simulink model "v13_aa_rii_16r128dr_ver104"  (Simulink 7.9)
settings: Solver=FixedStepDiscrete  SolverMode=SingleTasking  StartTime=0.0  StopTime=32768  RelTol=1e-3
undefined symbols (not defined in the file or in its folder): sfp
libraries: xbsIndex_r4 x19795, xps_library x54, casper_library_misc x36, dspsigops x32
(part 4 of 212)

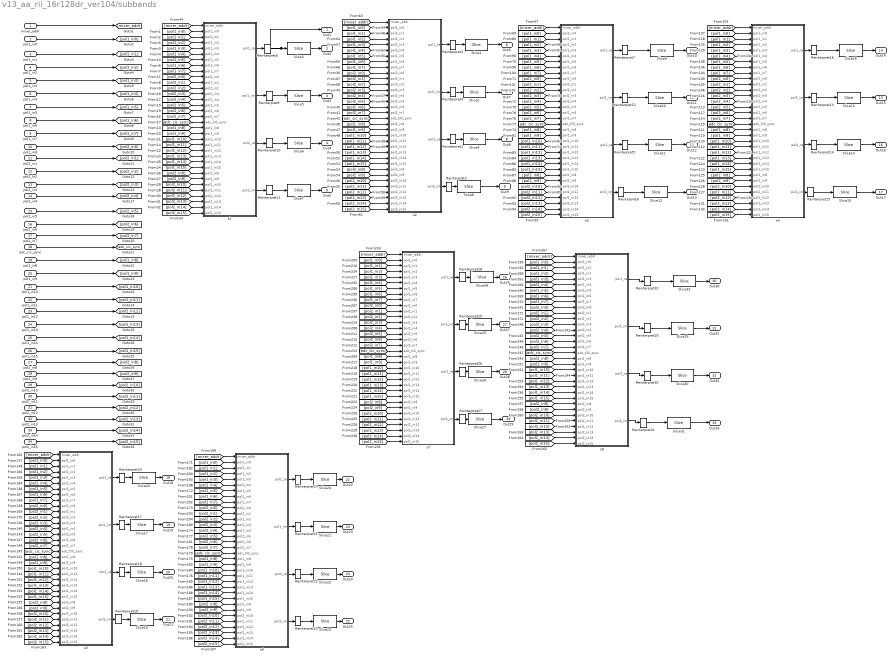
== system v13_aa_rii_16r128dr_ver104/subbands ==
  mixer_addr : Inport
  pol1_in0 : Inport  Port=2
  pol1_in1 : Inport  Port=3
  pol1_in2 : Inport  Port=4
  pol1_in3 : Inport  Port=5
  pol1_in4 : Inport  Port=6
  pol1_in5 : Inport  Port=7
  pol1_in6 : Inport  Port=8
  pol1_in7 : Inport  Port=9
  pol2_in0 : Inport  Port=10
  pol2_in1 : Inport  Port=11
  pol2_In2 : Inport  Port=12
  pol2_in3 : Inport  Port=13
  pol2_in4 : Inport  Port=14
  pol2_in5 : Inport  Port=15
  pol2_in6 : Inport  Port=16
  pol2_in7 : Inport  Port=17
  adc_cic_sync : Inport  Port=18
  pol1_in8 : Inport  Port=19
  pol1_in9 : Inport  Port=20
  pol1_in10 : Inport  Port=21
  pol1_in11 : Inport  Port=22
  pol1_in12 : Inport  Port=23
  pol1_in13 : Inport  Port=24
  pol1_in14 : Inport  Port=25
  pol1_in15 : Inport  Port=26
  pol2_in8 : Inport  Port=27
  pol2_in9 : Inport  Port=28
  pol2_in10 : Inport  Port=29
  pol2_in11 : Inport  Port=30
  pol2_in12 : Inport  Port=31
  pol2_in13 : Inport  Port=32
  pol2_in14 : Inport  Port=33
  pol2_in15 : Inport  Port=34
  Reinterpret{1..32} (32 alike) : xbsIndex_r4/Reinterpret  force_arith_type=on  arith_type=Unsigned  force_bin_pt=on  bin_pt=0  block_type=reinterpret
  Slice{1..32} (32 alike) : xbsIndex_r4/Slice  nbits=8  boolean_output=off  mode=Upper Bit Location + Width  bit1=0  base1=MSB of Input  bit0=0  base0=LSB of Input  dbl_ovrd=off  block_type=slice
  s1 : Subsystem  mask{mult_latency=5, add_latency=2, bram_length=10}
      mask prompts: mult_latency "Multiplier Latency"; add_latency "Adder Latency"; bram_length "BRAM Length(2^n)"
  s2 : Subsystem  mask{mult_latency=5, add_latency=2, bram_length=10}
      mask prompts: mult_latency "Multiplier Latency"; add_latency "Adder Latency"; bram_length "BRAM Length(2^n)"
  s3 : Subsystem  mask{mult_latency=5, add_latency=2, bram_length=10}
      mask prompts: mult_latency "Multiplier Latency"; add_latency "Adder Latency"; bram_length "BRAM Length(2^n)"
  s4 : Subsystem  mask{mult_latency=5, add_latency=2, bram_length=10}
      mask prompts: mult_latency "Multiplier Latency"; add_latency "Adder Latency"; bram_length "BRAM Length(2^n)"
  s5 : Subsystem  mask{mult_latency=5, add_latency=2, bram_length=10}
      mask prompts: mult_latency "Multiplier Latency"; add_latency "Adder Latency"; bram_length "BRAM Length(2^n)"
  s6 : Subsystem  mask{mult_latency=5, add_latency=2, bram_length=10}
      mask prompts: mult_latency "Multiplier Latency"; add_latency "Adder Latency"; bram_length "BRAM Length(2^n)"
  s7 : Subsystem  mask{mult_latency=5, add_latency=2, bram_length=10}
      mask prompts: mult_latency "Multiplier Latency"; add_latency "Adder Latency"; bram_length "BRAM Length(2^n)"
  s8 : Subsystem  mask{mult_latency=5, add_latency=2, bram_length=10}
      mask prompts: mult_latency "Multiplier Latency"; add_latency "Adder Latency"; bram_length "BRAM Length(2^n)"
  Out1 : Outport
  Out2 : Outport  Port=2
  Out3 : Outport  Port=3
  Out4 : Outport  Port=4
  Out5 : Outport  Port=5
  Out6 : Outport  Port=6
  Out7 : Outport  Port=7
  Out8 : Outport  Port=8
  Out9 : Outport  Port=9
  Out10 : Outport  Port=10
  Out11 : Outport  Port=11
  Out12 : Outport  Port=12
  Out13 : Outport  Port=13
  Out14 : Outport  Port=14
  Out15 : Outport  Port=15
  Out16 : Outport  Port=16
  Out17 : Outport  Port=17
  Out18 : Outport  Port=18
  Out19 : Outport  Port=19
  Out20 : Outport  Port=20
  Out21 : Outport  Port=21
  Out22 : Outport  Port=22
  Out23 : Outport  Port=23
  Out24 : Outport  Port=24
  Out25 : Outport  Port=25
  Out26 : Outport  Port=26
  Out27 : Outport  Port=27
  Out28 : Outport  Port=28
  Out29 : Outport  Port=29
  Out30 : Outport  Port=30
  Out31 : Outport  Port=31
  Out32 : Outport  Port=32
  Out33 : Outport  Port=33
  wires:
  Reinterpret8/1 -> Slice3/1, Out1/1
  Reinterpret9/1 -> Slice5/1
  Reinterpret10/1 -> Slice6/1
  Reinterpret11/1 -> Slice7/1
  s1/1(pol1_re) -> Reinterpret8/1
  s1/2(pol1_im) -> Reinterpret9/1
  s1/3(pol2_re) -> Reinterpret10/1
  s1/4(pol2_im) -> Reinterpret11/1
  Slice3/1 -> Out2/1
  Slice5/1 -> Out3/1
  Slice6/1 -> Out4/1
  Slice7/1 -> Out5/1
  Reinterpret3/1 -> Slice1/1
  Reinterpret4/1 -> Slice2/1
  Reinterpret1/1 -> Slice4/1
  Reinterpret2/1 -> Slice8/1
  s2/1(pol1_re) -> Reinterpret3/1
  s2/2(pol1_im) -> Reinterpret4/1
  s2/3(pol2_re) -> Reinterpret1/1
  s2/4(pol2_im) -> Reinterpret2/1
  Slice8/1 -> Out9/1
  Slice1/1 -> Out6/1
  Slice2/1 -> Out7/1
  Slice4/1 -> Out8/1
  Reinterpret7/1 -> Slice9/1
  Reinterpret12/1 -> Slice10/1
  Reinterpret5/1 -> Slice11/1
  Reinterpret6/1 -> Slice12/1
  s3/1(pol1_re) -> Reinterpret7/1
  s3/2(pol1_im) -> Reinterpret12/1
  s3/3(pol2_re) -> Reinterpret5/1
  s3/4(pol2_im) -> Reinterpret6/1
  Slice12/1 -> Out13/1
  Slice9/1 -> Out10/1
  Slice10/1 -> Out11/1
  Slice11/1 -> Out12/1
  Reinterpret16/1 -> Slice16/1
  Reinterpret13/1 -> Slice13/1
  Reinterpret14/1 -> Slice14/1
  Reinterpret15/1 -> Slice15/1
  s4/1(pol1_re) -> Reinterpret16/1
  s4/2(pol1_im) -> Reinterpret13/1
  s4/3(pol2_re) -> Reinterpret14/1
  s4/4(pol2_im) -> Reinterpret15/1
  Slice15/1 -> Out17/1
  Slice16/1 -> Out14/1
  Slice13/1 -> Out15/1
  Slice14/1 -> Out16/1
  Reinterpret20/1 -> Slice20/1
  Reinterpret17/1 -> Slice17/1
  Reinterpret18/1 -> Slice18/1
  Reinterpret19/1 -> Slice19/1
  s5/1(pol1_re) -> Reinterpret20/1
  s5/2(pol1_im) -> Reinterpret17/1
  s5/3(pol2_re) -> Reinterpret18/1
  s5/4(pol2_im) -> Reinterpret19/1
  Slice19/1 -> Out21/1
  Slice20/1 -> Out18/1
  Slice17/1 -> Out19/1
  Slice18/1 -> Out20/1
  Reinterpret24/1 -> Slice24/1
  Reinterpret21/1 -> Slice21/1
  Reinterpret22/1 -> Slice22/1
  Reinterpret23/1 -> Slice23/1
  s6/1(pol1_re) -> Reinterpret24/1
  s6/2(pol1_im) -> Reinterpret21/1
  s6/3(pol2_re) -> Reinterpret22/1
  s6/4(pol2_im) -> Reinterpret23/1
  Slice23/1 -> Out25/1
  Slice24/1 -> Out22/1
  Slice21/1 -> Out23/1
  Slice22/1 -> Out24/1
  Reinterpret28/1 -> Slice28/1
  Reinterpret25/1 -> Slice25/1
  Reinterpret26/1 -> Slice26/1
  Reinterpret27/1 -> Slice27/1
  s7/1(pol1_re) -> Reinterpret28/1
  s7/2(pol1_im) -> Reinterpret25/1
  s7/3(pol2_re) -> Reinterpret26/1
  s7/4(pol2_im) -> Reinterpret27/1
  Slice27/1 -> Out29/1
  Slice28/1 -> Out26/1
  Slice25/1 -> Out27/1
  Slice26/1 -> Out28/1
  Reinterpret32/1 -> Slice32/1
  Reinterpret29/1 -> Slice29/1
  Reinterpret30/1 -> Slice30/1
  Reinterpret31/1 -> Slice31/1
  s8/1(pol1_re) -> Reinterpret32/1
  s8/2(pol1_im) -> Reinterpret29/1
  s8/3(pol2_re) -> Reinterpret30/1
  s8/4(pol2_im) -> Reinterpret31/1
  Slice31/1 -> Out33/1
  Slice32/1 -> Out30/1
  Slice29/1 -> Out31/1
  Slice30/1 -> Out32/1
  mixer_addr/1 -> [mixer_addr]
  pol1_in0/1 -> [pol1_in0]
  pol1_in1/1 -> [pol1_in1]
  pol1_in2/1 -> [pol1_in2]
  pol1_in3/1 -> [pol1_in3]
  pol1_in4/1 -> [pol1_in4]
  pol1_in5/1 -> [pol1_in5]
  pol1_in6/1 -> [pol1_in6]
  pol1_in7/1 -> [pol1_in7]
  pol2_in0/1 -> [pol2_in0]
  pol2_in1/1 -> [pol2_in1]
  pol2_In2/1 -> [pol2_in2]
  pol2_in3/1 -> [pol2_in3]
  pol2_in4/1 -> [pol2_in4]
  pol2_in5/1 -> [pol2_in5]
  pol2_in6/1 -> [pol2_in6]
  pol2_in7/1 -> [pol2_in7]
  adc_cic_sync/1 -> [adc_cic_sync]
  pol1_in8/1 -> [pol1_in8]
  pol1_in9/1 -> [pol1_in9]
  pol1_in10/1 -> [pol1_in10]
  pol1_in11/1 -> [pol1_in11]
  pol1_in12/1 -> [pol1_in12]
  pol1_in13/1 -> [pol1_in13]
  pol1_in14/1 -> [pol1_in14]
  pol1_in15/1 -> [pol1_in15]
  pol2_in8/1 -> [pol2_in8]
  pol2_in9/1 -> [pol2_in9]
  pol2_in10/1 -> [pol2_in10]
  pol2_in11/1 -> [pol2_in11]
  pol2_in12/1 -> [pol2_in12]
  pol2_in13/1 -> [pol2_in13]
  pol2_in14/1 -> [pol2_in14]
  pol2_in15/1 -> [pol2_in15]
  [mixer_addr] -> s1/1(mixer_addr)
  [pol1_in0] -> s1/2(pol1_in0)
  [pol1_in1] -> s1/3(pol1_in1)
  [pol1_in2] -> s1/4(pol1_in2)
  [pol1_in3] -> s1/5(pol1_in3)
  [pol1_in4] -> s1/6(pol1_in4)
  [pol1_in5] -> s1/7(pol1_in5)
  [pol1_in6] -> s1/8(pol1_in6)
  [pol1_in7] -> s1/9(pol1_in7)
  [pol2_in0] -> s1/10(pol2_in0)
  [pol2_in1] -> s1/11(pol2_in1)
  [pol2_in2] -> s1/12(pol2_in2)
  [pol2_in3] -> s1/13(pol2_in3)
  [pol2_in4] -> s1/14(pol2_in4)
  [pol2_in5] -> s1/15(pol2_in5)
  [pol2_in6] -> s1/16(pol2_in6)
  [pol2_in7] -> s1/17(pol2_in7)
  [adc_cic_sync] -> s1/18(adc_CIC_sync)
  [pol1_in8] -> s1/19(pol1_in8)
  [pol1_in9] -> s1/20(pol1_in9)
  [pol1_in10] -> s1/21(pol1_in10)
  [pol1_in11] -> s1/22(pol1_in11)
  [pol1_in12] -> s1/23(pol1_in12)
  [pol1_in13] -> s1/24(pol1_in13)
  [pol1_in14] -> s1/25(pol1_in14)
  [pol1_in15] -> s1/26(pol1_in15)
  [pol2_in8] -> s1/27(pol2_in8)
  [pol2_in9] -> s1/28(pol2_in9)
  [pol2_in10] -> s1/29(pol2_in10)
  [pol2_in11] -> s1/30(pol2_in11)
  [pol2_in12] -> s1/31(pol2_in12)
  [pol2_in13] -> s1/32(pol2_in13)
  [pol2_in14] -> s1/33(pol2_in14)
  [pol2_in15] -> s1/34(pol2_in15)
  [mixer_addr] -> s2/1(mixer_addr)
  [pol1_in0] -> s2/2(pol1_in0)
  [pol1_in1] -> s2/3(pol1_in1)
  [pol1_in2] -> s2/4(pol1_in2)
  [pol1_in3] -> s2/5(pol1_in3)
  [pol1_in4] -> s2/6(pol1_in4)
  [pol1_in5] -> s2/7(pol1_in5)
  [pol1_in6] -> s2/8(pol1_in6)
  [pol1_in7] -> s2/9(pol1_in7)
  [pol2_in0] -> s2/10(pol2_in0)
  [pol2_in1] -> s2/11(pol2_in1)
  [pol2_in2] -> s2/12(pol2_in2)
  [pol2_in3] -> s2/13(pol2_in3)
  [pol2_in4] -> s2/14(pol2_in4)
  [pol2_in5] -> s2/15(pol2_in5)
  [pol2_in6] -> s2/16(pol2_in6)
  [pol2_in7] -> s2/17(pol2_in7)
  [adc_cic_sync] -> s2/18(adc_CIC_sync)
  [pol1_in8] -> s2/19(pol1_in8)
  [pol1_in9] -> s2/20(pol1_in9)
  [pol1_in10] -> s2/21(pol1_in10)
  [pol1_in11] -> s2/22(pol1_in11)
  [pol1_in12] -> s2/23(pol1_in12)
  [pol1_in13] -> s2/24(pol1_in13)
  [pol1_in14] -> s2/25(pol1_in14)
  [pol1_in15] -> s2/26(pol1_in15)
  [pol2_in8] -> s2/27(pol2_in8)
  [pol2_in9] -> s2/28(pol2_in9)
  [pol2_in10] -> s2/29(pol2_in10)
  [pol2_in11] -> s2/30(pol2_in11)
  [pol2_in12] -> s2/31(pol2_in12)
  [pol2_in13] -> s2/32(pol2_in13)
  [pol2_in14] -> s2/33(pol2_in14)
  [pol2_in15] -> s2/34(pol2_in15)
  [mixer_addr] -> s3/1(mixer_addr)
  [pol1_in0] -> s3/2(pol1_in0)
  [pol1_in1] -> s3/3(pol1_in1)
  [pol1_in2] -> s3/4(pol1_in2)
  [pol1_in3] -> s3/5(pol1_in3)
  [pol1_in4] -> s3/6(pol1_in4)
  [pol1_in5] -> s3/7(pol1_in5)
  [pol1_in6] -> s3/8(pol1_in6)
  [pol1_in7] -> s3/9(pol1_in7)
  [pol2_in0] -> s3/10(pol2_in0)
  [pol2_in1] -> s3/11(pol2_in1)
  [pol2_in2] -> s3/12(pol2_in2)
  [pol2_in3] -> s3/13(pol2_in3)
  [pol2_in4] -> s3/14(pol2_in4)
  [pol2_in5] -> s3/15(pol2_in5)
  [pol2_in6] -> s3/16(pol2_in6)
  [pol2_in7] -> s3/17(pol2_in7)
  [adc_cic_sync] -> s3/18(adc_CIC_sync)
  [pol1_in8] -> s3/19(pol1_in8)
  [pol1_in9] -> s3/20(pol1_in9)
  [pol1_in10] -> s3/21(pol1_in10)
  [pol1_in11] -> s3/22(pol1_in11)
  [pol1_in12] -> s3/23(pol1_in12)
  [pol1_in13] -> s3/24(pol1_in13)
  [pol1_in14] -> s3/25(pol1_in14)
  [pol1_in15] -> s3/26(pol1_in15)
  [pol2_in8] -> s3/27(pol2_in8)
  [pol2_in9] -> s3/28(pol2_in9)
  [pol2_in10] -> s3/29(pol2_in10)
  [pol2_in11] -> s3/30(pol2_in11)
  [pol2_in12] -> s3/31(pol2_in12)
  [pol2_in13] -> s3/32(pol2_in13)
  [pol2_in14] -> s3/33(pol2_in14)
  [pol2_in15] -> s3/34(pol2_in15)
  [mixer_addr] -> s4/1(mixer_addr)
  [pol1_in0] -> s4/2(pol1_in0)
  [pol1_in1] -> s4/3(pol1_in1)
  [pol1_in2] -> s4/4(pol1_in2)
  [pol1_in3] -> s4/5(pol1_in3)
  [pol1_in4] -> s4/6(pol1_in4)
  [pol1_in5] -> s4/7(pol1_in5)
  [pol1_in6] -> s4/8(pol1_in6)
  [pol1_in7] -> s4/9(pol1_in7)
  [pol2_in0] -> s4/10(pol2_in0)
  [pol2_in1] -> s4/11(pol2_in1)
  [pol2_in2] -> s4/12(pol2_in2)
  [pol2_in3] -> s4/13(pol2_in3)
  [pol2_in4] -> s4/14(pol2_in4)
  [pol2_in5] -> s4/15(pol2_in5)
  [pol2_in6] -> s4/16(pol2_in6)
  [pol2_in7] -> s4/17(pol2_in7)
  [adc_cic_sync] -> s4/18(adc_CIC_sync)
  [pol1_in8] -> s4/19(pol1_in8)
  [pol1_in9] -> s4/20(pol1_in9)
  [pol1_in10] -> s4/21(pol1_in10)
  [pol1_in11] -> s4/22(pol1_in11)
  [pol1_in12] -> s4/23(pol1_in12)
  [pol1_in13] -> s4/24(pol1_in13)
  [pol1_in14] -> s4/25(pol1_in14)
  [pol1_in15] -> s4/26(pol1_in15)
  [pol2_in8] -> s4/27(pol2_in8)
  [pol2_in9] -> s4/28(pol2_in9)
  [pol2_in10] -> s4/29(pol2_in10)
  [pol2_in11] -> s4/30(pol2_in11)
  [pol2_in12] -> s4/31(pol2_in12)
  [pol2_in13] -> s4/32(pol2_in13)
  [pol2_in14] -> s4/33(pol2_in14)
  [pol2_in15] -> s4/34(pol2_in15)
  [mixer_addr] -> s5/1(mixer_addr)
  [pol1_in0] -> s5/2(pol1_in0)
  [pol1_in1] -> s5/3(pol1_in1)
  [pol1_in2] -> s5/4(pol1_in2)
  [pol1_in3] -> s5/5(pol1_in3)
  [pol1_in4] -> s5/6(pol1_in4)
  [pol1_in5] -> s5/7(pol1_in5)
  [pol1_in6] -> s5/8(pol1_in6)
  [pol1_in7] -> s5/9(pol1_in7)
  [pol2_in0] -> s5/10(pol2_in0)
  [pol2_in1] -> s5/11(pol2_in1)
  [pol2_in2] -> s5/12(pol2_in2)
  [pol2_in3] -> s5/13(pol2_in3)
  [pol2_in4] -> s5/14(pol2_in4)
  [pol2_in5] -> s5/15(pol2_in5)
  [pol2_in6] -> s5/16(pol2_in6)
  [pol2_in7] -> s5/17(pol2_in7)
  [adc_cic_sync] -> s5/18(adc_CIC_sync)
  [pol1_in8] -> s5/19(pol1_in8)
  [pol1_in9] -> s5/20(pol1_in9)
  [pol1_in10] -> s5/21(pol1_in10)
  [pol1_in11] -> s5/22(pol1_in11)
  [pol1_in12] -> s5/23(pol1_in12)
  [pol1_in13] -> s5/24(pol1_in13)
  [pol1_in14] -> s5/25(pol1_in14)
  [pol1_in15] -> s5/26(pol1_in15)
  [pol2_in8] -> s5/27(pol2_in8)
  [pol2_in9] -> s5/28(pol2_in9)
  [pol2_in10] -> s5/29(pol2_in10)
  [pol2_in11] -> s5/30(pol2_in11)
  [pol2_in12] -> s5/31(pol2_in12)
  [pol2_in13] -> s5/32(pol2_in13)
  [pol2_in14] -> s5/33(pol2_in14)
  [pol2_in15] -> s5/34(pol2_in15)
  [mixer_addr] -> s8/1(mixer_addr)
  [mixer_addr] -> s7/1(mixer_addr)
  [mixer_addr] -> s6/1(mixer_addr)
  [pol1_in0] -> s6/2(pol1_in0)
  [pol1_in1] -> s6/3(pol1_in1)
  [pol1_in2] -> s6/4(pol1_in2)
  [pol1_in3] -> s6/5(pol1_in3)
  [pol1_in4] -> s6/6(pol1_in4)
  [pol1_in5] -> s6/7(pol1_in5)
  [pol1_in6] -> s6/8(pol1_in6)
  [pol1_in7] -> s6/9(pol1_in7)
  [pol2_in0] -> s6/10(pol2_in0)
  [pol2_in1] -> s6/11(pol2_in1)
  [pol2_in2] -> s6/12(pol2_in2)
  [pol2_in3] -> s6/13(pol2_in3)
  [pol2_in4] -> s6/14(pol2_in4)
  [pol2_in5] -> s6/15(pol2_in5)
  [pol2_in6] -> s6/16(pol2_in6)
  [pol2_in7] -> s6/17(pol2_in7)
  [adc_cic_sync] -> s6/18(adc_CIC_sync)
  [pol1_in8] -> s6/19(pol1_in8)
  [pol1_in9] -> s6/20(pol1_in9)
  [pol1_in10] -> s6/21(pol1_in10)
  [pol1_in11] -> s6/22(pol1_in11)
  [pol1_in12] -> s6/23(pol1_in12)
  [pol1_in13] -> s6/24(pol1_in13)
  [pol1_in14] -> s6/25(pol1_in14)
  [pol1_in15] -> s6/26(pol1_in15)
  [pol2_in8] -> s6/27(pol2_in8)
  [pol2_in9] -> s6/28(pol2_in9)
  [pol2_in10] -> s6/29(pol2_in10)
  [pol2_in11] -> s6/30(pol2_in11)
  [pol2_in12] -> s6/31(pol2_in12)
  [pol2_in13] -> s6/32(pol2_in13)
  [pol2_in14] -> s6/33(pol2_in14)
  [pol2_in15] -> s6/34(pol2_in15)
  [pol1_in0] -> s7/2(pol1_in0)
  [pol1_in1] -> s7/3(pol1_in1)
  [pol1_in2] -> s7/4(pol1_in2)
  [pol1_in3] -> s7/5(pol1_in3)
  [pol1_in4] -> s7/6(pol1_in4)
  [pol1_in5] -> s7/7(pol1_in5)
  [pol1_in6] -> s7/8(pol1_in6)
  [pol1_in7] -> s7/9(pol1_in7)
  [pol2_in0] -> s7/10(pol2_in0)
  [pol2_in1] -> s7/11(pol2_in1)
  [pol2_in2] -> s7/12(pol2_in2)
  [pol2_in3] -> s7/13(pol2_in3)
  [pol2_in4] -> s7/14(pol2_in4)
  [pol2_in5] -> s7/15(pol2_in5)
  [pol2_in6] -> s7/16(pol2_in6)
  [pol2_in7] -> s7/17(pol2_in7)
  [adc_cic_sync] -> s7/18(adc_CIC_sync)
  [pol1_in8] -> s7/19(pol1_in8)
  [pol1_in9] -> s7/20(pol1_in9)
  [pol1_in10] -> s7/21(pol1_in10)
  [pol1_in11] -> s7/22(pol1_in11)
  [pol1_in12] -> s7/23(pol1_in12)
  [pol1_in13] -> s7/24(pol1_in13)
  [pol1_in14] -> s7/25(pol1_in14)
  [pol1_in15] -> s7/26(pol1_in15)
  [pol2_in8] -> s7/27(pol2_in8)
  [pol2_in9] -> s7/28(pol2_in9)
  [pol2_in10] -> s7/29(pol2_in10)
  [pol2_in11] -> s7/30(pol2_in11)
  [pol2_in12] -> s7/31(pol2_in12)
  [pol2_in13] -> s7/32(pol2_in13)
  [pol2_in14] -> s7/33(pol2_in14)
  [pol2_in15] -> s7/34(pol2_in15)
  [pol1_in0] -> s8/2(pol1_in0)
  [pol1_in1] -> s8/3(pol1_in1)
  [pol1_in2] -> s8/4(pol1_in2)
  [pol1_in3] -> s8/5(pol1_in3)
  [pol1_in4] -> s8/6(pol1_in4)
  [pol1_in5] -> s8/7(pol1_in5)
  [pol1_in6] -> s8/8(pol1_in6)
  [pol1_in7] -> s8/9(pol1_in7)
  [pol2_in0] -> s8/10(pol2_in0)
  [pol2_in1] -> s8/11(pol2_in1)
  [pol2_in2] -> s8/12(pol2_in2)
  [pol2_in3] -> s8/13(pol2_in3)
  [pol2_in4] -> s8/14(pol2_in4)
  [pol2_in5] -> s8/15(pol2_in5)
  [pol2_in6] -> s8/16(pol2_in6)
  [pol2_in7] -> s8/17(pol2_in7)
  [adc_cic_sync] -> s8/18(adc_CIC_sync)
  [pol1_in8] -> s8/19(pol1_in8)
  [pol1_in9] -> s8/20(pol1_in9)
  [pol1_in10] -> s8/21(pol1_in10)
  [pol1_in11] -> s8/22(pol1_in11)
  [pol1_in12] -> s8/23(pol1_in12)
  [pol1_in13] -> s8/24(pol1_in13)
  [pol1_in14] -> s8/25(pol1_in14)
  [pol1_in15] -> s8/26(pol1_in15)
  [pol2_in8] -> s8/27(pol2_in8)
  [pol2_in9] -> s8/28(pol2_in9)
  [pol2_in10] -> s8/29(pol2_in10)
  [pol2_in11] -> s8/30(pol2_in11)
  [pol2_in12] -> s8/31(pol2_in12)
  [pol2_in13] -> s8/32(pol2_in13)
  [pol2_in14] -> s8/33(pol2_in14)
  [pol2_in15] -> s8/34(pol2_in15)

== system v13_aa_rii_16r128dr_ver104/s32_cicp1re/bram ==
  addr : Inport
  data_in : Inport  Port=2
  we : Inport  Port=3
  calc_add : Subsystem
  convert_addr : xbsIndex_r4/Convert  gui_display_data_type=Fixed-point  arith_type=Unsigned  n_bits=addr_width  bin_pt=0  float_type=Single  exp_bits=8  fraction_bits=24  quantization=Truncate  overflow=Wrap  en=off  latency=0  dbl_ovrd=off  pipeline=off  block_type=convert
      settings: xl_area=[0, 0, 0, 0, 0, 0, 0]
  convert_din : xbsIndex_r4/Convert  gui_display_data_type=Fixed-point  arith_type=Unsigned  n_bits=data_width  bin_pt=data_bin_pt  float_type=Single  exp_bits=8  fraction_bits=24  quantization=Truncate  overflow=Wrap  en=off  latency=0  dbl_ovrd=off  pipeline=off  block_type=convert
      settings: xl_area=[0, 0, 0, 0, 0, 0, 0]
  convert_din1 : xbsIndex_r4/Convert  gui_display_data_type=Fixed-point  arith_type=Unsigned  n_bits=data_width  bin_pt=data_bin_pt  float_type=Single  exp_bits=8  fraction_bits=24  quantization=Truncate  overflow=Wrap  en=off  latency=0  dbl_ovrd=off  pipeline=off  block_type=convert
      settings: xl_area=[0, 0, 0, 0, 0, 0, 0]
  convert_we : xbsIndex_r4/Convert  gui_display_data_type=Fixed-point  arith_type=Unsigned  n_bits=1  bin_pt=0  float_type=Single  exp_bits=8  fraction_bits=24  quantization=Truncate  overflow=Wrap  en=off  latency=0  dbl_ovrd=off  pipeline=off  block_type=convert
      settings: xl_area=[0, 0, 0, 0, 0, 0, 0]
  mem : Subsystem
  munge_in : Subsystem  AncestorBlock=casper_library_flow_control/munge  mask(munge){divisions=1, div_size=32, order=[0]}
      mask prompts: divisions "Number of divisions"; div_size "Division size (bits)"; order "Division packing order"
      mask help: Splits an input bus into the specified number of divisions and then recombines them in the order specified (most significant division = 0,least = number divisions-1)
      mask init:
      | munge_init(gcb, ...
      |     'divisions', divisions, ...
      |     'div_size', div_size, ...
      |     'order', order);
      settings: AttributesFormatString=|
      | [0]
      | 32 bits
  munge_out : Subsystem  AncestorBlock=casper_library_flow_control/munge  mask(munge){divisions=1, div_size=32, order=[0]}
      mask prompts: divisions "Number of divisions"; div_size "Division size (bits)"; order "Division packing order"
      mask help: Splits an input bus into the specified number of divisions and then recombines them in the order specified (most significant division = 0,least = number divisions-1)
      mask init:
      | munge_init(gcb, ...
      |     'divisions', divisions, ...
      |     'div_size', div_size, ...
      |     'order', order);
      settings: AttributesFormatString=|
      | [0]
      | 32 bits
  v13_aa_rii_16r128dr_ver104_s32_cicp1re_bram_addr : xbsIndex_r4/Gateway Out  inherit_from_input=off  hdl_port=on  timing_constraint=None  locs_specified=off  LOCs={'A5','B9','A9','C1','A3','G6','E9','A6','A7','A8','B8','B1','B3','D9','B5','B4','B6','C2'}  UseAsDAC=off  DACChannel='1'  block_type=gatewayout
      settings: infoedit="Gateway out block.  Converts Xilinx fixed point inputs into ouputs of type Simulink integer, double, or fixed point.<P><P>Hardware notes:  In hardware these blocks become top level output ports or are discarded, depending on how they are configured."  xl_area=[0, 0, 0, 0, 0, 0, 0]  sginterface={'Nallatech'=>{'xdspkit'=>{'non_memory_mapped_port'=>'uprev_Shared_BRAM_addr'}},'Xilinx'=>{'jtaghwcosim'=>{'non_memory_mapped_port'=>'uprev_Shared_BRAM_addr'},'xdspkit'=>{'non_memory_mapped_port'=>'uprev_Shared_BRAM_addr'}}}
  v13_aa_rii_16r128dr_ver104_s32_cicp1re_bram_data_in : xbsIndex_r4/Gateway Out  inherit_from_input=off  hdl_port=on  timing_constraint=None  locs_specified=off  LOCs={'B21','B22','A22','B23','B19','C19','A20','A21','A23','B24','A24','A25','E18','D18','C18','B18'}  UseAsDAC=off  DACChannel='1'  block_type=gatewayout
      settings: infoedit="Gateway out block.  Converts Xilinx fixed point inputs into ouputs of type Simulink integer, double, or fixed point.<P><P>Hardware notes:  In hardware these blocks become top level output ports or are discarded, depending on how they are configured."  xl_area=[0, 0, 0, 0, 0, 0, 0]  sginterface={'Nallatech'=>{'xdspkit'=>{'non_memory_mapped_port'=>'uprev_Shared_BRAM_data_in'}},'Xilinx'=>{'jtaghwcosim'=>{'non_memory_mapped_port'=>'uprev_Shared_BRAM_data_in'},'xdspkit'=>{'non_memory_mapped_port'=>'uprev_Shared_BRAM_data_in'}}}
  v13_aa_rii_16r128dr_ver104_s32_cicp1re_bram_data_out : xbsIndex_r4/Gateway In  gui_display_data_type=Fixed-point  arith_type=Unsigned  n_bits=data_width  bin_pt=data_bin_pt  preci_type=Single  exp_width=8  frac_width=24  quantization=Truncate  overflow=Wrap  period=sample_rate  dbl_ovrd=off  timing_constraint=None  locs_specified=off  LOCs={}  UseAsADC=off  ADCChannel='1'  inherit_from_input=off  hdl_port=on  block_type=gatewayin
      settings: infoedit="Gateway in block.  Converts inputs of type Simulink integer, double and fixed point to  Xilinx fixed point type.<br><br>Hardware notes:  In hardware these blocks become top level input ports."  xl_area=[0, 0, 0, 0, 0, 0, 0]
  v13_aa_rii_16r128dr_ver104_s32_cicp1re_bram_we : xbsIndex_r4/Gateway Out  inherit_from_input=off  hdl_port=on  timing_constraint=None  locs_specified=off  LOCs={'A14'}  UseAsDAC=off  DACChannel='1'  block_type=gatewayout
      settings: infoedit="Gateway out block.  Converts Xilinx fixed point inputs into ouputs of type Simulink integer, double, or fixed point.<P><P>Hardware notes:  In hardware these blocks become top level output ports or are discarded, depending on how they are configured."  xl_area=[0, 0, 0, 0, 0, 0, 0]
  data_out : Outport
  wires:
  convert_din/1 -> munge_in/1(din)
  convert_din1/1 -> v13_aa_rii_16r128dr_ver104_s32_cicp1re_bram_data_in/1
  convert_addr/1 -> v13_aa_rii_16r128dr_ver104_s32_cicp1re_bram_addr/1
  calc_add/1(out) -> convert_addr/1
  munge_out/1(dout) -> data_out/1
  munge_in/1(dout) -> convert_din1/1
  v13_aa_rii_16r128dr_ver104_s32_cicp1re_bram_we/1 -> mem/3(In3)
  v13_aa_rii_16r128dr_ver104_s32_cicp1re_bram_data_in/1 -> mem/2(In2)
  v13_aa_rii_16r128dr_ver104_s32_cicp1re_bram_addr/1 -> mem/1(In1)
  convert_we/1 -> v13_aa_rii_16r128dr_ver104_s32_cicp1re_bram_we/1
  we/1 -> convert_we/1
  addr/1 -> calc_add/1(in)
  v13_aa_rii_16r128dr_ver104_s32_cicp1re_bram_data_out/1 -> munge_out/1(din)
  data_in/1 -> convert_din/1
  mem/1(Out1) -> v13_aa_rii_16r128dr_ver104_s32_cicp1re_bram_data_out/1

== system v13_aa_rii_16r128dr_ver104/s32_s1p1im/bram ==
  addr : Inport
  data_in : Inport  Port=2
  we : Inport  Port=3
  calc_add : Subsystem
  convert_addr : xbsIndex_r4/Convert  gui_display_data_type=Fixed-point  arith_type=Unsigned  n_bits=addr_width  bin_pt=0  float_type=Single  exp_bits=8  fraction_bits=24  quantization=Truncate  overflow=Wrap  en=off  latency=0  dbl_ovrd=off  pipeline=off  block_type=convert
      settings: xl_area=[0, 0, 0, 0, 0, 0, 0]
  convert_din : xbsIndex_r4/Convert  gui_display_data_type=Fixed-point  arith_type=Unsigned  n_bits=data_width  bin_pt=data_bin_pt  float_type=Single  exp_bits=8  fraction_bits=24  quantization=Truncate  overflow=Wrap  en=off  latency=0  dbl_ovrd=off  pipeline=off  block_type=convert
      settings: xl_area=[0, 0, 0, 0, 0, 0, 0]
  convert_din1 : xbsIndex_r4/Convert  gui_display_data_type=Fixed-point  arith_type=Unsigned  n_bits=data_width  bin_pt=data_bin_pt  float_type=Single  exp_bits=8  fraction_bits=24  quantization=Truncate  overflow=Wrap  en=off  latency=0  dbl_ovrd=off  pipeline=off  block_type=convert
      settings: xl_area=[0, 0, 0, 0, 0, 0, 0]
  convert_we : xbsIndex_r4/Convert  gui_display_data_type=Fixed-point  arith_type=Unsigned  n_bits=1  bin_pt=0  float_type=Single  exp_bits=8  fraction_bits=24  quantization=Truncate  overflow=Wrap  en=off  latency=0  dbl_ovrd=off  pipeline=off  block_type=convert
      settings: xl_area=[0, 0, 0, 0, 0, 0, 0]
  mem : Subsystem
  munge_in : Subsystem  AncestorBlock=casper_library_flow_control/munge  mask(munge){divisions=1, div_size=32, order=[0]}
      mask prompts: divisions "Number of divisions"; div_size "Division size (bits)"; order "Division packing order"
      mask help: Splits an input bus into the specified number of divisions and then recombines them in the order specified (most significant division = 0,least = number divisions-1)
      mask init:
      | munge_init(gcb, ...
      |     'divisions', divisions, ...
      |     'div_size', div_size, ...
      |     'order', order);
      settings: AttributesFormatString=|
      | [0]
      | 32 bits
  munge_out : Subsystem  AncestorBlock=casper_library_flow_control/munge  mask(munge){divisions=1, div_size=32, order=[0]}
      mask prompts: divisions "Number of divisions"; div_size "Division size (bits)"; order "Division packing order"
      mask help: Splits an input bus into the specified number of divisions and then recombines them in the order specified (most significant division = 0,least = number divisions-1)
      mask init:
      | munge_init(gcb, ...
      |     'divisions', divisions, ...
      |     'div_size', div_size, ...
      |     'order', order);
      settings: AttributesFormatString=|
      | [0]
      | 32 bits
  v13_aa_rii_16r128dr_ver104_s32_s1p1im_bram_addr : xbsIndex_r4/Gateway Out  inherit_from_input=off  hdl_port=on  timing_constraint=None  locs_specified=off  LOCs={'A5','B9','A9','C1','A3','G6','E9','A6','A7','A8','B8','B1','B3','D9','B5','B4','B6','C2'}  UseAsDAC=off  DACChannel='1'  block_type=gatewayout
      settings: infoedit="Gateway out block.  Converts Xilinx fixed point inputs into ouputs of type Simulink integer, double, or fixed point.<P><P>Hardware notes:  In hardware these blocks become top level output ports or are discarded, depending on how they are configured."  xl_area=[0, 0, 0, 0, 0, 0, 0]  sginterface={'Nallatech'=>{'xdspkit'=>{'non_memory_mapped_port'=>'uprev_Shared_BRAM_addr'}},'Xilinx'=>{'jtaghwcosim'=>{'non_memory_mapped_port'=>'uprev_Shared_BRAM_addr'},'xdspkit'=>{'non_memory_mapped_port'=>'uprev_Shared_BRAM_addr'}}}
  v13_aa_rii_16r128dr_ver104_s32_s1p1im_bram_data_in : xbsIndex_r4/Gateway Out  inherit_from_input=off  hdl_port=on  timing_constraint=None  locs_specified=off  LOCs={'B21','B22','A22','B23','B19','C19','A20','A21','A23','B24','A24','A25','E18','D18','C18','B18'}  UseAsDAC=off  DACChannel='1'  block_type=gatewayout
      settings: infoedit="Gateway out block.  Converts Xilinx fixed point inputs into ouputs of type Simulink integer, double, or fixed point.<P><P>Hardware notes:  In hardware these blocks become top level output ports or are discarded, depending on how they are configured."  xl_area=[0, 0, 0, 0, 0, 0, 0]  sginterface={'Nallatech'=>{'xdspkit'=>{'non_memory_mapped_port'=>'uprev_Shared_BRAM_data_in'}},'Xilinx'=>{'jtaghwcosim'=>{'non_memory_mapped_port'=>'uprev_Shared_BRAM_data_in'},'xdspkit'=>{'non_memory_mapped_port'=>'uprev_Shared_BRAM_data_in'}}}
  v13_aa_rii_16r128dr_ver104_s32_s1p1im_bram_data_out : xbsIndex_r4/Gateway In  gui_display_data_type=Fixed-point  arith_type=Unsigned  n_bits=data_width  bin_pt=data_bin_pt  preci_type=Single  exp_width=8  frac_width=24  quantization=Truncate  overflow=Wrap  period=sample_rate  dbl_ovrd=off  timing_constraint=None  locs_specified=off  LOCs={}  UseAsADC=off  ADCChannel='1'  inherit_from_input=off  hdl_port=on  block_type=gatewayin
      settings: infoedit="Gateway in block.  Converts inputs of type Simulink integer, double and fixed point to  Xilinx fixed point type.<br><br>Hardware notes:  In hardware these blocks become top level input ports."  xl_area=[0, 0, 0, 0, 0, 0, 0]
  v13_aa_rii_16r128dr_ver104_s32_s1p1im_bram_we : xbsIndex_r4/Gateway Out  inherit_from_input=off  hdl_port=on  timing_constraint=None  locs_specified=off  LOCs={'A14'}  UseAsDAC=off  DACChannel='1'  block_type=gatewayout
      settings: infoedit="Gateway out block.  Converts Xilinx fixed point inputs into ouputs of type Simulink integer, double, or fixed point.<P><P>Hardware notes:  In hardware these blocks become top level output ports or are discarded, depending on how they are configured."  xl_area=[0, 0, 0, 0, 0, 0, 0]
  data_out : Outport
  wires:
  convert_din/1 -> munge_in/1(din)
  convert_din1/1 -> v13_aa_rii_16r128dr_ver104_s32_s1p1im_bram_data_in/1
  convert_addr/1 -> v13_aa_rii_16r128dr_ver104_s32_s1p1im_bram_addr/1
  calc_add/1(out) -> convert_addr/1
  munge_out/1(dout) -> data_out/1
  munge_in/1(dout) -> convert_din1/1
  v13_aa_rii_16r128dr_ver104_s32_s1p1im_bram_we/1 -> mem/3(In3)
  v13_aa_rii_16r128dr_ver104_s32_s1p1im_bram_data_in/1 -> mem/2(In2)
  v13_aa_rii_16r128dr_ver104_s32_s1p1im_bram_addr/1 -> mem/1(In1)
  convert_we/1 -> v13_aa_rii_16r128dr_ver104_s32_s1p1im_bram_we/1
  we/1 -> convert_we/1
  addr/1 -> calc_add/1(in)
  v13_aa_rii_16r128dr_ver104_s32_s1p1im_bram_data_out/1 -> munge_out/1(din)
  data_in/1 -> convert_din/1
  mem/1(Out1) -> v13_aa_rii_16r128dr_ver104_s32_s1p1im_bram_data_out/1

== system v13_aa_rii_16r128dr_ver104/s32_s1p1re/bram ==
  addr : Inport
  data_in : Inport  Port=2
  we : Inport  Port=3
  calc_add : Subsystem
  convert_addr : xbsIndex_r4/Convert  gui_display_data_type=Fixed-point  arith_type=Unsigned  n_bits=addr_width  bin_pt=0  float_type=Single  exp_bits=8  fraction_bits=24  quantization=Truncate  overflow=Wrap  en=off  latency=0  dbl_ovrd=off  pipeline=off  block_type=convert
      settings: xl_area=[0, 0, 0, 0, 0, 0, 0]
  convert_din : xbsIndex_r4/Convert  gui_display_data_type=Fixed-point  arith_type=Unsigned  n_bits=data_width  bin_pt=data_bin_pt  float_type=Single  exp_bits=8  fraction_bits=24  quantization=Truncate  overflow=Wrap  en=off  latency=0  dbl_ovrd=off  pipeline=off  block_type=convert
      settings: xl_area=[0, 0, 0, 0, 0, 0, 0]
  convert_din1 : xbsIndex_r4/Convert  gui_display_data_type=Fixed-point  arith_type=Unsigned  n_bits=data_width  bin_pt=data_bin_pt  float_type=Single  exp_bits=8  fraction_bits=24  quantization=Truncate  overflow=Wrap  en=off  latency=0  dbl_ovrd=off  pipeline=off  block_type=convert
      settings: xl_area=[0, 0, 0, 0, 0, 0, 0]
  convert_we : xbsIndex_r4/Convert  gui_display_data_type=Fixed-point  arith_type=Unsigned  n_bits=1  bin_pt=0  float_type=Single  exp_bits=8  fraction_bits=24  quantization=Truncate  overflow=Wrap  en=off  latency=0  dbl_ovrd=off  pipeline=off  block_type=convert
      settings: xl_area=[0, 0, 0, 0, 0, 0, 0]
  mem : Subsystem
  munge_in : Subsystem  AncestorBlock=casper_library_flow_control/munge  mask(munge){divisions=1, div_size=32, order=[0]}
      mask prompts: divisions "Number of divisions"; div_size "Division size (bits)"; order "Division packing order"
      mask help: Splits an input bus into the specified number of divisions and then recombines them in the order specified (most significant division = 0,least = number divisions-1)
      mask init:
      | munge_init(gcb, ...
      |     'divisions', divisions, ...
      |     'div_size', div_size, ...
      |     'order', order);
      settings: AttributesFormatString=|
      | [0]
      | 32 bits
  munge_out : Subsystem  AncestorBlock=casper_library_flow_control/munge  mask(munge){divisions=1, div_size=32, order=[0]}
      mask prompts: divisions "Number of divisions"; div_size "Division size (bits)"; order "Division packing order"
      mask help: Splits an input bus into the specified number of divisions and then recombines them in the order specified (most significant division = 0,least = number divisions-1)
      mask init:
      | munge_init(gcb, ...
      |     'divisions', divisions, ...
      |     'div_size', div_size, ...
      |     'order', order);
      settings: AttributesFormatString=|
      | [0]
      | 32 bits
  v13_aa_rii_16r128dr_ver104_s32_s1p1re_bram_addr : xbsIndex_r4/Gateway Out  inherit_from_input=off  hdl_port=on  timing_constraint=None  locs_specified=off  LOCs={'A5','B9','A9','C1','A3','G6','E9','A6','A7','A8','B8','B1','B3','D9','B5','B4','B6','C2'}  UseAsDAC=off  DACChannel='1'  block_type=gatewayout
      settings: infoedit="Gateway out block.  Converts Xilinx fixed point inputs into ouputs of type Simulink integer, double, or fixed point.<P><P>Hardware notes:  In hardware these blocks become top level output ports or are discarded, depending on how they are configured."  xl_area=[0, 0, 0, 0, 0, 0, 0]  sginterface={'Nallatech'=>{'xdspkit'=>{'non_memory_mapped_port'=>'uprev_Shared_BRAM_addr'}},'Xilinx'=>{'jtaghwcosim'=>{'non_memory_mapped_port'=>'uprev_Shared_BRAM_addr'},'xdspkit'=>{'non_memory_mapped_port'=>'uprev_Shared_BRAM_addr'}}}
  v13_aa_rii_16r128dr_ver104_s32_s1p1re_bram_data_in : xbsIndex_r4/Gateway Out  inherit_from_input=off  hdl_port=on  timing_constraint=None  locs_specified=off  LOCs={'B21','B22','A22','B23','B19','C19','A20','A21','A23','B24','A24','A25','E18','D18','C18','B18'}  UseAsDAC=off  DACChannel='1'  block_type=gatewayout
      settings: infoedit="Gateway out block.  Converts Xilinx fixed point inputs into ouputs of type Simulink integer, double, or fixed point.<P><P>Hardware notes:  In hardware these blocks become top level output ports or are discarded, depending on how they are configured."  xl_area=[0, 0, 0, 0, 0, 0, 0]  sginterface={'Nallatech'=>{'xdspkit'=>{'non_memory_mapped_port'=>'uprev_Shared_BRAM_data_in'}},'Xilinx'=>{'jtaghwcosim'=>{'non_memory_mapped_port'=>'uprev_Shared_BRAM_data_in'},'xdspkit'=>{'non_memory_mapped_port'=>'uprev_Shared_BRAM_data_in'}}}
  v13_aa_rii_16r128dr_ver104_s32_s1p1re_bram_data_out : xbsIndex_r4/Gateway In  gui_display_data_type=Fixed-point  arith_type=Unsigned  n_bits=data_width  bin_pt=data_bin_pt  preci_type=Single  exp_width=8  frac_width=24  quantization=Truncate  overflow=Wrap  period=sample_rate  dbl_ovrd=off  timing_constraint=None  locs_specified=off  LOCs={}  UseAsADC=off  ADCChannel='1'  inherit_from_input=off  hdl_port=on  block_type=gatewayin
      settings: infoedit="Gateway in block.  Converts inputs of type Simulink integer, double and fixed point to  Xilinx fixed point type.<br><br>Hardware notes:  In hardware these blocks become top level input ports."  xl_area=[0, 0, 0, 0, 0, 0, 0]
  v13_aa_rii_16r128dr_ver104_s32_s1p1re_bram_we : xbsIndex_r4/Gateway Out  inherit_from_input=off  hdl_port=on  timing_constraint=None  locs_specified=off  LOCs={'A14'}  UseAsDAC=off  DACChannel='1'  block_type=gatewayout
      settings: infoedit="Gateway out block.  Converts Xilinx fixed point inputs into ouputs of type Simulink integer, double, or fixed point.<P><P>Hardware notes:  In hardware these blocks become top level output ports or are discarded, depending on how they are configured."  xl_area=[0, 0, 0, 0, 0, 0, 0]
  data_out : Outport
  wires:
  convert_din/1 -> munge_in/1(din)
  convert_din1/1 -> v13_aa_rii_16r128dr_ver104_s32_s1p1re_bram_data_in/1
  convert_addr/1 -> v13_aa_rii_16r128dr_ver104_s32_s1p1re_bram_addr/1
  calc_add/1(out) -> convert_addr/1
  munge_out/1(dout) -> data_out/1
  munge_in/1(dout) -> convert_din1/1
  v13_aa_rii_16r128dr_ver104_s32_s1p1re_bram_we/1 -> mem/3(In3)
  v13_aa_rii_16r128dr_ver104_s32_s1p1re_bram_data_in/1 -> mem/2(In2)
  v13_aa_rii_16r128dr_ver104_s32_s1p1re_bram_addr/1 -> mem/1(In1)
  convert_we/1 -> v13_aa_rii_16r128dr_ver104_s32_s1p1re_bram_we/1
  we/1 -> convert_we/1
  addr/1 -> calc_add/1(in)
  v13_aa_rii_16r128dr_ver104_s32_s1p1re_bram_data_out/1 -> munge_out/1(din)
  data_in/1 -> convert_din/1
  mem/1(Out1) -> v13_aa_rii_16r128dr_ver104_s32_s1p1re_bram_data_out/1

== system v13_aa_rii_16r128dr_ver104/s64_adcp1/bram_lsb ==
  addr : Inport
  data_in : Inport  Port=2
  we : Inport  Port=3
  calc_add : Subsystem
  convert_addr : xbsIndex_r4/Convert  gui_display_data_type=Fixed-point  arith_type=Unsigned  n_bits=addr_width  bin_pt=0  float_type=Single  exp_bits=8  fraction_bits=24  quantization=Truncate  overflow=Wrap  en=off  latency=0  dbl_ovrd=off  pipeline=off  block_type=convert
      settings: xl_area=[0, 0, 0, 0, 0, 0, 0]
  convert_din : xbsIndex_r4/Convert  gui_display_data_type=Fixed-point  arith_type=Unsigned  n_bits=data_width  bin_pt=data_bin_pt  float_type=Single  exp_bits=8  fraction_bits=24  quantization=Truncate  overflow=Wrap  en=off  latency=0  dbl_ovrd=off  pipeline=off  block_type=convert
      settings: xl_area=[0, 0, 0, 0, 0, 0, 0]
  convert_din1 : xbsIndex_r4/Convert  gui_display_data_type=Fixed-point  arith_type=Unsigned  n_bits=data_width  bin_pt=data_bin_pt  float_type=Single  exp_bits=8  fraction_bits=24  quantization=Truncate  overflow=Wrap  en=off  latency=0  dbl_ovrd=off  pipeline=off  block_type=convert
      settings: xl_area=[0, 0, 0, 0, 0, 0, 0]
  convert_we : xbsIndex_r4/Convert  gui_display_data_type=Fixed-point  arith_type=Unsigned  n_bits=1  bin_pt=0  float_type=Single  exp_bits=8  fraction_bits=24  quantization=Truncate  overflow=Wrap  en=off  latency=0  dbl_ovrd=off  pipeline=off  block_type=convert
      settings: xl_area=[0, 0, 0, 0, 0, 0, 0]
  mem : Subsystem
  munge_in : Subsystem  AncestorBlock=casper_library_flow_control/munge  mask(munge){divisions=1, div_size=32, order=[0]}
      mask prompts: divisions "Number of divisions"; div_size "Division size (bits)"; order "Division packing order"
      mask help: Splits an input bus into the specified number of divisions and then recombines them in the order specified (most significant division = 0,least = number divisions-1)
      mask init:
      | munge_init(gcb, ...
      |     'divisions', divisions, ...
      |     'div_size', div_size, ...
      |     'order', order);
      settings: AttributesFormatString=|
      | [0]
      | 32 bits
  munge_out : Subsystem  AncestorBlock=casper_library_flow_control/munge  mask(munge){divisions=1, div_size=32, order=[0]}
      mask prompts: divisions "Number of divisions"; div_size "Division size (bits)"; order "Division packing order"
      mask help: Splits an input bus into the specified number of divisions and then recombines them in the order specified (most significant division = 0,least = number divisions-1)
      mask init:
      | munge_init(gcb, ...
      |     'divisions', divisions, ...
      |     'div_size', div_size, ...
      |     'order', order);
      settings: AttributesFormatString=|
      | [0]
      | 32 bits
  v13_aa_rii_16r128dr_ver104_s64_adcp1_bram_lsb_addr : xbsIndex_r4/Gateway Out  inherit_from_input=off  hdl_port=on  timing_constraint=None  locs_specified=off  LOCs={'A5','B9','A9','C1','A3','G6','E9','A6','A7','A8','B8','B1','B3','D9','B5','B4','B6','C2'}  UseAsDAC=off  DACChannel='1'  block_type=gatewayout
      settings: infoedit="Gateway out block.  Converts Xilinx fixed point inputs into ouputs of type Simulink integer, double, or fixed point.<P><P>Hardware notes:  In hardware these blocks become top level output ports or are discarded, depending on how they are configured."  xl_area=[0, 0, 0, 0, 0, 0, 0]  sginterface={'Nallatech'=>{'xdspkit'=>{'non_memory_mapped_port'=>'uprev_Shared_BRAM_addr'}},'Xilinx'=>{'jtaghwcosim'=>{'non_memory_mapped_port'=>'uprev_Shared_BRAM_addr'},'xdspkit'=>{'non_memory_mapped_port'=>'uprev_Shared_BRAM_addr'}}}
  v13_aa_rii_16r128dr_ver104_s64_adcp1_bram_lsb_data_in : xbsIndex_r4/Gateway Out  inherit_from_input=off  hdl_port=on  timing_constraint=None  locs_specified=off  LOCs={'B21','B22','A22','B23','B19','C19','A20','A21','A23','B24','A24','A25','E18','D18','C18','B18'}  UseAsDAC=off  DACChannel='1'  block_type=gatewayout
      settings: infoedit="Gateway out block.  Converts Xilinx fixed point inputs into ouputs of type Simulink integer, double, or fixed point.<P><P>Hardware notes:  In hardware these blocks become top level output ports or are discarded, depending on how they are configured."  xl_area=[0, 0, 0, 0, 0, 0, 0]  sginterface={'Nallatech'=>{'xdspkit'=>{'non_memory_mapped_port'=>'uprev_Shared_BRAM_data_in'}},'Xilinx'=>{'jtaghwcosim'=>{'non_memory_mapped_port'=>'uprev_Shared_BRAM_data_in'},'xdspkit'=>{'non_memory_mapped_port'=>'uprev_Shared_BRAM_data_in'}}}
  v13_aa_rii_16r128dr_ver104_s64_adcp1_bram_lsb_data_out : xbsIndex_r4/Gateway In  gui_display_data_type=Fixed-point  arith_type=Unsigned  n_bits=data_width  bin_pt=data_bin_pt  preci_type=Single  exp_width=8  frac_width=24  quantization=Truncate  overflow=Wrap  period=sample_rate  dbl_ovrd=off  timing_constraint=None  locs_specified=off  LOCs={}  UseAsADC=off  ADCChannel='1'  inherit_from_input=off  hdl_port=on  block_type=gatewayin
      settings: infoedit="Gateway in block.  Converts inputs of type Simulink integer, double and fixed point to  Xilinx fixed point type.<br><br>Hardware notes:  In hardware these blocks become top level input ports."  xl_area=[0, 0, 0, 0, 0, 0, 0]
  v13_aa_rii_16r128dr_ver104_s64_adcp1_bram_lsb_we : xbsIndex_r4/Gateway Out  inherit_from_input=off  hdl_port=on  timing_constraint=None  locs_specified=off  LOCs={'A14'}  UseAsDAC=off  DACChannel='1'  block_type=gatewayout
      settings: infoedit="Gateway out block.  Converts Xilinx fixed point inputs into ouputs of type Simulink integer, double, or fixed point.<P><P>Hardware notes:  In hardware these blocks become top level output ports or are discarded, depending on how they are configured."  xl_area=[0, 0, 0, 0, 0, 0, 0]
  data_out : Outport
  wires:
  convert_din/1 -> munge_in/1(din)
  convert_din1/1 -> v13_aa_rii_16r128dr_ver104_s64_adcp1_bram_lsb_data_in/1
  convert_addr/1 -> v13_aa_rii_16r128dr_ver104_s64_adcp1_bram_lsb_addr/1
  calc_add/1(out) -> convert_addr/1
  munge_out/1(dout) -> data_out/1
  munge_in/1(dout) -> convert_din1/1
  v13_aa_rii_16r128dr_ver104_s64_adcp1_bram_lsb_we/1 -> mem/3(In3)
  v13_aa_rii_16r128dr_ver104_s64_adcp1_bram_lsb_data_in/1 -> mem/2(In2)
  v13_aa_rii_16r128dr_ver104_s64_adcp1_bram_lsb_addr/1 -> mem/1(In1)
  convert_we/1 -> v13_aa_rii_16r128dr_ver104_s64_adcp1_bram_lsb_we/1
  we/1 -> convert_we/1
  addr/1 -> calc_add/1(in)
  v13_aa_rii_16r128dr_ver104_s64_adcp1_bram_lsb_data_out/1 -> munge_out/1(din)
  data_in/1 -> convert_din/1
  mem/1(Out1) -> v13_aa_rii_16r128dr_ver104_s64_adcp1_bram_lsb_data_out/1

== system v13_aa_rii_16r128dr_ver104/s64_adcp1/bram_msb ==
  addr : Inport
  data_in : Inport  Port=2
  we : Inport  Port=3
  calc_add : Subsystem
  convert_addr : xbsIndex_r4/Convert  gui_display_data_type=Fixed-point  arith_type=Unsigned  n_bits=addr_width  bin_pt=0  float_type=Single  exp_bits=8  fraction_bits=24  quantization=Truncate  overflow=Wrap  en=off  latency=0  dbl_ovrd=off  pipeline=off  block_type=convert
      settings: xl_area=[0, 0, 0, 0, 0, 0, 0]
  convert_din : xbsIndex_r4/Convert  gui_display_data_type=Fixed-point  arith_type=Unsigned  n_bits=data_width  bin_pt=data_bin_pt  float_type=Single  exp_bits=8  fraction_bits=24  quantization=Truncate  overflow=Wrap  en=off  latency=0  dbl_ovrd=off  pipeline=off  block_type=convert
      settings: xl_area=[0, 0, 0, 0, 0, 0, 0]
  convert_din1 : xbsIndex_r4/Convert  gui_display_data_type=Fixed-point  arith_type=Unsigned  n_bits=data_width  bin_pt=data_bin_pt  float_type=Single  exp_bits=8  fraction_bits=24  quantization=Truncate  overflow=Wrap  en=off  latency=0  dbl_ovrd=off  pipeline=off  block_type=convert
      settings: xl_area=[0, 0, 0, 0, 0, 0, 0]
  convert_we : xbsIndex_r4/Convert  gui_display_data_type=Fixed-point  arith_type=Unsigned  n_bits=1  bin_pt=0  float_type=Single  exp_bits=8  fraction_bits=24  quantization=Truncate  overflow=Wrap  en=off  latency=0  dbl_ovrd=off  pipeline=off  block_type=convert
      settings: xl_area=[0, 0, 0, 0, 0, 0, 0]
  mem : Subsystem
  munge_in : Subsystem  AncestorBlock=casper_library_flow_control/munge  mask(munge){divisions=1, div_size=32, order=[0]}
      mask prompts: divisions "Number of divisions"; div_size "Division size (bits)"; order "Division packing order"
      mask help: Splits an input bus into the specified number of divisions and then recombines them in the order specified (most significant division = 0,least = number divisions-1)
      mask init:
      | munge_init(gcb, ...
      |     'divisions', divisions, ...
      |     'div_size', div_size, ...
      |     'order', order);
      settings: AttributesFormatString=|
      | [0]
      | 32 bits
  munge_out : Subsystem  AncestorBlock=casper_library_flow_control/munge  mask(munge){divisions=1, div_size=32, order=[0]}
      mask prompts: divisions "Number of divisions"; div_size "Division size (bits)"; order "Division packing order"
      mask help: Splits an input bus into the specified number of divisions and then recombines them in the order specified (most significant division = 0,least = number divisions-1)
      mask init:
      | munge_init(gcb, ...
      |     'divisions', divisions, ...
      |     'div_size', div_size, ...
      |     'order', order);
      settings: AttributesFormatString=|
      | [0]
      | 32 bits
  v13_aa_rii_16r128dr_ver104_s64_adcp1_bram_msb_addr : xbsIndex_r4/Gateway Out  inherit_from_input=off  hdl_port=on  timing_constraint=None  locs_specified=off  LOCs={'A5','B9','A9','C1','A3','G6','E9','A6','A7','A8','B8','B1','B3','D9','B5','B4','B6','C2'}  UseAsDAC=off  DACChannel='1'  block_type=gatewayout
      settings: infoedit="Gateway out block.  Converts Xilinx fixed point inputs into ouputs of type Simulink integer, double, or fixed point.<P><P>Hardware notes:  In hardware these blocks become top level output ports or are discarded, depending on how they are configured."  xl_area=[0, 0, 0, 0, 0, 0, 0]  sginterface={'Nallatech'=>{'xdspkit'=>{'non_memory_mapped_port'=>'uprev_Shared_BRAM_addr'}},'Xilinx'=>{'jtaghwcosim'=>{'non_memory_mapped_port'=>'uprev_Shared_BRAM_addr'},'xdspkit'=>{'non_memory_mapped_port'=>'uprev_Shared_BRAM_addr'}}}
  v13_aa_rii_16r128dr_ver104_s64_adcp1_bram_msb_data_in : xbsIndex_r4/Gateway Out  inherit_from_input=off  hdl_port=on  timing_constraint=None  locs_specified=off  LOCs={'B21','B22','A22','B23','B19','C19','A20','A21','A23','B24','A24','A25','E18','D18','C18','B18'}  UseAsDAC=off  DACChannel='1'  block_type=gatewayout
      settings: infoedit="Gateway out block.  Converts Xilinx fixed point inputs into ouputs of type Simulink integer, double, or fixed point.<P><P>Hardware notes:  In hardware these blocks become top level output ports or are discarded, depending on how they are configured."  xl_area=[0, 0, 0, 0, 0, 0, 0]  sginterface={'Nallatech'=>{'xdspkit'=>{'non_memory_mapped_port'=>'uprev_Shared_BRAM_data_in'}},'Xilinx'=>{'jtaghwcosim'=>{'non_memory_mapped_port'=>'uprev_Shared_BRAM_data_in'},'xdspkit'=>{'non_memory_mapped_port'=>'uprev_Shared_BRAM_data_in'}}}
  v13_aa_rii_16r128dr_ver104_s64_adcp1_bram_msb_data_out : xbsIndex_r4/Gateway In  gui_display_data_type=Fixed-point  arith_type=Unsigned  n_bits=data_width  bin_pt=data_bin_pt  preci_type=Single  exp_width=8  frac_width=24  quantization=Truncate  overflow=Wrap  period=sample_rate  dbl_ovrd=off  timing_constraint=None  locs_specified=off  LOCs={}  UseAsADC=off  ADCChannel='1'  inherit_from_input=off  hdl_port=on  block_type=gatewayin
      settings: infoedit="Gateway in block.  Converts inputs of type Simulink integer, double and fixed point to  Xilinx fixed point type.<br><br>Hardware notes:  In hardware these blocks become top level input ports."  xl_area=[0, 0, 0, 0, 0, 0, 0]
  v13_aa_rii_16r128dr_ver104_s64_adcp1_bram_msb_we : xbsIndex_r4/Gateway Out  inherit_from_input=off  hdl_port=on  timing_constraint=None  locs_specified=off  LOCs={'A14'}  UseAsDAC=off  DACChannel='1'  block_type=gatewayout
      settings: infoedit="Gateway out block.  Converts Xilinx fixed point inputs into ouputs of type Simulink integer, double, or fixed point.<P><P>Hardware notes:  In hardware these blocks become top level output ports or are discarded, depending on how they are configured."  xl_area=[0, 0, 0, 0, 0, 0, 0]
  data_out : Outport
  wires:
  convert_din/1 -> munge_in/1(din)
  convert_din1/1 -> v13_aa_rii_16r128dr_ver104_s64_adcp1_bram_msb_data_in/1
  convert_addr/1 -> v13_aa_rii_16r128dr_ver104_s64_adcp1_bram_msb_addr/1
  calc_add/1(out) -> convert_addr/1
  munge_out/1(dout) -> data_out/1
  munge_in/1(dout) -> convert_din1/1
  v13_aa_rii_16r128dr_ver104_s64_adcp1_bram_msb_we/1 -> mem/3(In3)
  v13_aa_rii_16r128dr_ver104_s64_adcp1_bram_msb_data_in/1 -> mem/2(In2)
  v13_aa_rii_16r128dr_ver104_s64_adcp1_bram_msb_addr/1 -> mem/1(In1)
  convert_we/1 -> v13_aa_rii_16r128dr_ver104_s64_adcp1_bram_msb_we/1
  we/1 -> convert_we/1
  addr/1 -> calc_add/1(in)
  v13_aa_rii_16r128dr_ver104_s64_adcp1_bram_msb_data_out/1 -> munge_out/1(din)
  data_in/1 -> convert_din/1
  mem/1(Out1) -> v13_aa_rii_16r128dr_ver104_s64_adcp1_bram_msb_data_out/1
